simulink model "mcspi_external_read_write_am263x"  (R2024b)
settings: Solver=FixedStepAuto  StartTime=0.0  StopTime=10.0  RelTol=1e-3  EnableMultiTasking=on
libraries: peripheral_library_blocks_am263x x6
settings Model: Creator=a0499511  LastModifiedBy=a1255762
settings OptimizationCC: DefaultParameterBehavior=Inlined  EnhancedBackFolding=on  PassReuseOutputArgsAs=Individual arguments  ZeroExternalMemoryAtStartup=off  ZeroInternalMemoryAtStartup=off  MaxStackSize=64  OptimizeBlockOrder=speed  DifferentSizesBufferReuse=on  OptimizationCustomize=off  EfficientTunableParamExpr=on
settings DebuggingCC: ParameterTunabilityLossMsg=error
settings HardwareCC: ProdBitPerPointer=32  ProdBitPerSizeT=32  ProdBitPerPtrDiffT=32  ProdLargestAtomicInteger=Long  ProdLargestAtomicFloat=Double  ProdWordSize=32  ProdLongLongMode=on  ProdHWDeviceType=ARM Compatible->ARM Cortex
settings SFSimCC: MATLABDynamicMemAlloc=off
settings RTWCC: Description=Embedded Coder  SystemTargetFile=ert.tlc  HardwareBoard=AM263X LP  TemplateMakefile=ert_default_tmf  Toolchain=TI ARM Clang Compiler AM263X | gmake
settings CodeAppCC: ForceParamTrailComments=on  IgnoreCustomStorageClasses=off  MaxIdLength=256  OperatorAnnotations=on  SimulinkDataObjDesc=on  SFDataObjDesc=on  InsertBlockDesc=on
settings ERTTargetCC: SupportVariableSizeSignals=on  ExtModeTransport=3  ExtModeStaticAlloc=on  ExtModeMexFile=ext_xcp  ExtModeIntrfLevel=Level2 - Open  ERTCustomFileTemplate=AM263x_codertarget_file_process.tlc
settings ConfigComp: CovBoundaryAbsTol=1.0e-5

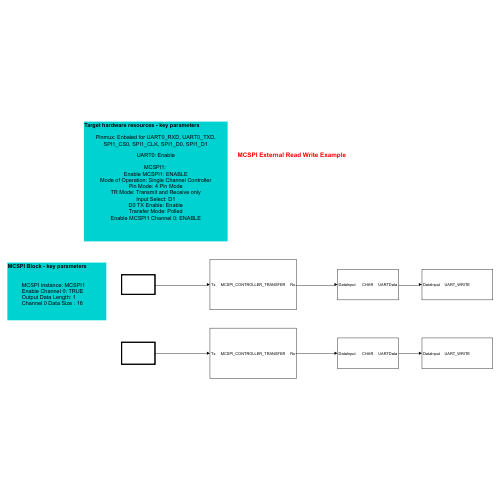
[thumbnail: Simulink's stored preview of the top-level diagram]
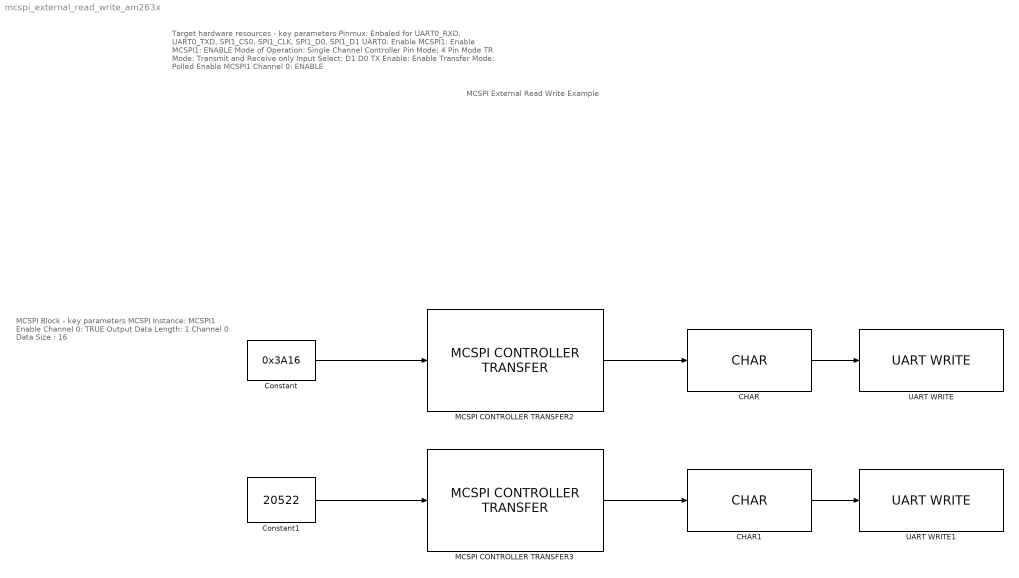
== system mcspi_external_read_write_am263x ==
  note: Target hardware resources - key parameters
      |
      | Pinmux: Enbaled for UART0_RXD, UART0_TXD, SPI1_CS0, SPI1_CLK, SPI1_D0, SPI1_D1
      |
      | UART0: Enable
      |
      | MCSPI1:
      | Enable MCSPI1: ENABLE
      | Mode of Operation: Single Channel Controller
      | Pin Mode: 4 Pin Mode
      | TR Mode: Transmit and Receive only
      | Input Select: D1
      | D0 TX Enable: Enable
      | Transfer Mode: Polled
      | Enable MCSPI1 Channel 0: ENABLE
  note: MCSPI External Read Write Example
  note: MCSPI Block - key parameters
      |
      | MCSPI Instance: MCSPI1
      | Enable Channel 0: TRUE
      | Output Data Length: 1
      | Channel 0 Data Size : 16
  CHAR : peripheral_library_blocks_am263x/CHAR
      settings: SourceProductName=Embedded Coder Support Package for AM263X - Peripheral Blocks
  CHAR1 : peripheral_library_blocks_am263x/CHAR
      settings: SourceProductName=Embedded Coder Support Package for AM263X - Peripheral Blocks
  Constant : Constant  Value=0x3A16  OutDataTypeStr=uint16  SampleTime=-1
  Constant1 : Constant  Value=20522  OutDataTypeStr=uint16  SampleTime=-1
  MCSPI CONTROLLER TRANSFER2 : peripheral_library_blocks_am263x/MCSPI CONTROLLER TRANSFER  MCSPI_Instance=MCSPI1  Enable_Channel0=on  Enable_Channel1=off  Output_Data_Length=1  Channel0_Data_Size=16  Channel0_Auto_CSDeassert=on  Channel1_Data_Size=8  Channel1_Auto_CSDeassert=on
      settings: SourceProductName=Embedded Coder Support Package for AM263X - Peripheral Blocks
  MCSPI CONTROLLER TRANSFER3 : peripheral_library_blocks_am263x/MCSPI CONTROLLER TRANSFER  MCSPI_Instance=MCSPI1  Enable_Channel0=on  Enable_Channel1=off  Output_Data_Length=1  Channel0_Data_Size=16  Channel0_Auto_CSDeassert=on  Channel1_Data_Size=8  Channel1_Auto_CSDeassert=on
      settings: SourceProductName=Embedded Coder Support Package for AM263X - Peripheral Blocks
  UART WRITE : peripheral_library_blocks_am263x/UART WRITE  UARTPort=UART0
      settings: SourceProductName=Embedded Coder Support Package for AM263X - Peripheral Blocks
  UART WRITE1 : peripheral_library_blocks_am263x/UART WRITE  UARTPort=UART0
      settings: SourceProductName=Embedded Coder Support Package for AM263X - Peripheral Blocks
  wires:
  Constant/1 -> MCSPI CONTROLLER TRANSFER2/1
  MCSPI CONTROLLER TRANSFER3/1 -> CHAR1/1
  CHAR1/1 -> UART WRITE1/1
  Constant1/1 -> MCSPI CONTROLLER TRANSFER3/1
  MCSPI CONTROLLER TRANSFER2/1 -> CHAR/1
  CHAR/1 -> UART WRITE/1
